simulink model "PrkgClimaEveMgr"  (R2022b)
settings: Solver=FixedStepDiscrete  StartTime=0.0  StopTime=10.0  FixedStep=0.2  RelTol=1e-3
(part 1 of 2)
settings Model: Creator=blue.ge  LastModifiedBy=blue.ge
settings DataIOCC: SaveFormat=StructureWithTime
settings OptimizationCC: DefaultParameterBehavior=Inlined  EnhancedBackFolding=on  PassReuseOutputArgsAs=Individual arguments  ZeroExternalMemoryAtStartup=off  ZeroInternalMemoryAtStartup=off  OptimizeBlockOrder=speed  DifferentSizesBufferReuse=on  OptimizationCustomize=off  EfficientTunableParamExpr=on
settings DebuggingCC: ParameterTunabilityLossMsg=error
settings ModelReferenceCC: ModelReferenceNumInstancesAllowed=Single
settings SFSimCC: MATLABDynamicMemAlloc=off
settings RTWCC: Description=AUTOSAR  SystemTargetFile=autosar.tlc  GenCodeOnly=on  TemplateMakefile=ert_default_tmf
settings CodeAppCC: ForceParamTrailComments=on  IgnoreCustomStorageClasses=off  OperatorAnnotations=on  SimulinkDataObjDesc=on  SFDataObjDesc=on  InsertBlockDesc=on
settings STFCustomTargetCC: TargetLangStandard=C99 (ISO)  MultiwordLength=256  ParMdlRefBuildCompliant=on  CompOptLevelCompliant=on  IncludeMdlTerminateFcn=off  CombineOutputUpdateFcns=on  SuppressErrorStatus=on  ERTCustomFileBanners=on  SupportNonFinite=off  SupportComplex=off  SupportContinuousTime=off  SupportNonInlinedSFcns=off  RemoveResetFunc=on  MATLABClassNameForMDSCustomization=Simulink.SoftwareTarget.ERTCustomization  AutosarCompliant=on  UseToolchainInfoCompliant=on  LookupTableObjectStructAxisOrder=2,1,3,4,...  LUTObjectStructOrderEvenSpacing=Size,Table,Breakpoints  SystemTargetFile=autosar.tlc
settings ERTTargetCC: TargetLangStandard=C99 (ISO)  ConcurrentExecutionCompliant=off  IncludeMdlTerminateFcn=off  SuppressErrorStatus=on  SupportNonFinite=off  SupportComplex=off  ModelStepFunctionPrototypeControlCompliant=off  CPPClassGenCompliant=off  AutosarCompliant=on  LookupTableObjectStructAxisOrder=2,1,3,4,...  LUTObjectStructOrderEvenSpacing=Size,Table,Breakpoints  InlinedParameterPlacement=NonHierarchical  ERTCustomFileTemplate=example_file_process.tlc

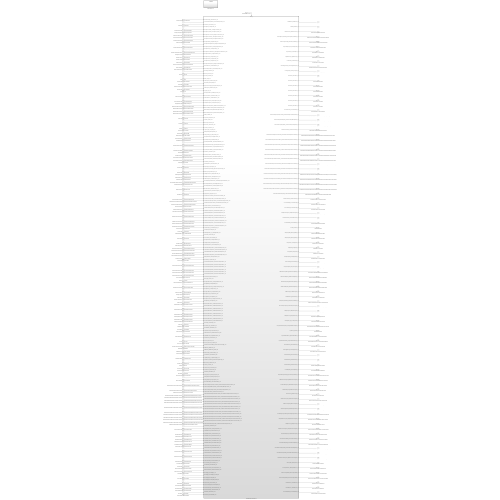
[thumbnail: Simulink's stored preview of the top-level diagram]
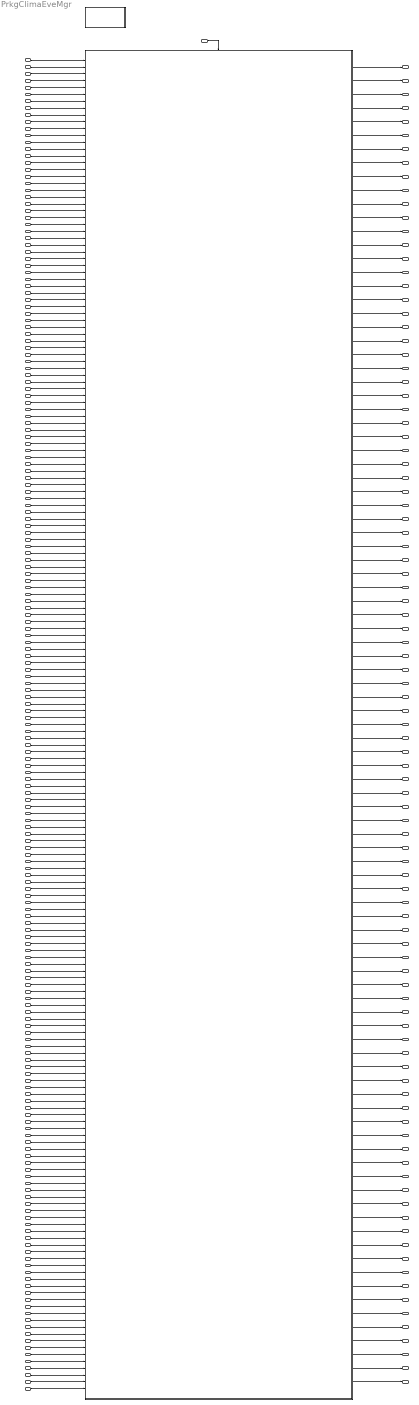
== system PrkgClimaEveMgr ==
  PrkgClimaEveMgr_Runnable : Inport  SampleTime=0.2  OutputFunctionCall=on
  AmbTFildForClimaNgcc_AmbTFildForClima : Inport  Port=2  OutDataTypeStr=T30  PortDimensions=1
  BookTrvlClimaActvReqfromExsrc_BookTrvlClimaActvReqfromExsrc : Inport  Port=3  OutDataTypeStr=Enum: OnOffNoReq  PortDimensions=1
  CarTiIntForClima_CarTiIntForClima : Inport  Port=4  OutDataTypeStr=CarTiGlb  PortDimensions=1
  ClimaCfgForCds1_ClimaCfgForCds1 : Inport  Port=5  OutDataTypeStr=Boolean  PortDimensions=1
  ClimaCfgForClimaCtrlMan1_ClimaCfgForClimaCtrlMan1 : Inport  Port=6  OutDataTypeStr=Boolean  PortDimensions=1
  ClimaCfgForCoolgPreCdn1_ClimaCfgForCoolgPreCdn1 : Inport  Port=7  OutDataTypeStr=Enum: ClimaCfgForCoolgPreCdn1  PortDimensions=1
  ClimaCfgForCooltCircForClima1_ClimaCfgForCooltCircForClima1 : Inport  Port=8  OutDataTypeStr=Boolean  PortDimensions=1
  ClimaCfgForEcuCooltVlvOutp1_ClimaCfgForEcuCooltVlvOutp1 : Inport  Port=9  OutDataTypeStr=Enum: ClimaCfgForEcuCooltVlvOutp1  PortDimensions=1
  ClimaCfgForExtVentnClimaCtrl_ClimaCfgForExtVentnClimaCtrl : Inport  Port=10  OutDataTypeStr=Enum: CarCfgForExtVentnClimaCtrl  PortDimensions=1
  ClimaCfgForFoldCSD_ClimaCfgForFoldCSD : Inport  Port=11  OutDataTypeStr=Boolean  PortDimensions=1
  ClimaCfgForForRemEngStrtPreCdn_ClimaCfgForForRemEngStrtPreCdn : Inport  Port=12  OutDataTypeStr=Enum: CarCfgForRemEngStrtPreCdn  PortDimensions=1
  ClimaCfgForHeatgPreCdnAvl1_ClimaCfgForHeatgPreCdnAvl1 : Inport  Port=13  OutDataTypeStr=Boolean  PortDimensions=1
  ClimaCfgForHeatrTyp1_ClimaCfgForHeatrTyp1 : Inport  Port=14  OutDataTypeStr=Enum: ClimaCfgForHeatrTyp1  PortDimensions=1
  ClimaCfgForHvacHeatrElecFrntPrsnt1_ClimaCfgForHvacHeatrElecFrntPrsnt1 : Inport  Port=15  OutDataTypeStr=Boolean  PortDimensions=1
  ClimaCfgForPostCdnAvl1_ClimaCfgForPostCdnAvl1 : Inport  Port=16  OutDataTypeStr=Boolean  PortDimensions=1
  ClimaCfgForPreClng1_ClimaCfgForPreClng1 : Inport  Port=17  OutDataTypeStr=Boolean  PortDimensions=1
  ClimaCfgForVehElec1_ClimaCfgForVehElec1 : Inport  Port=18  OutDataTypeStr=Boolean  PortDimensions=1
  ClimaCfgForVehHyb1_ClimaCfgForVehHyb1 : Inport  Port=19  OutDataTypeStr=Boolean  PortDimensions=1
  ClimaCfgForVrntOfVehSysElec1_ClimaCfgForVrntOfVehSysElec1 : Inport  Port=20  OutDataTypeStr=Enum: ClimaCenCfgForVrntOfVehSysElec1  PortDimensions=1
  ClimaOvrHeatProWarn_ClimaOvrHeatProWarn : Inport  Port=21  OutDataTypeStr=Enum: ClimaOvrheatProWarnSts  PortDimensions=1
  ClimaOvrHeatReqFromExrsc_ClimaOvrHeatReqFromExrsc : Inport  Port=22  OutDataTypeStr=Enum: PreCdngReqFromExtSrc  PortDimensions=1
  ClimaStrtTiDly_ClimaStrtTiDly : Inport  Port=23  OutDataTypeStr=Ti39  PortDimensions=1
  ClimaTmrSts_ClimaTmrSts : Inport  Port=24  OutDataTypeStr=Enum: OnOff1  PortDimensions=1
  CmptmtClsd_CmptmtClsd : Inport  Port=25  OutDataTypeStr=Boolean  PortDimensions=1
  COHSts_COHSts : Inport  Port=26  OutDataTypeStr=Enum: CabinOHSts  PortDimensions=1
  CooltTForPrkgClima_CooltTForPrkgClima : Inport  Port=27  OutDataTypeStr=T30  PortDimensions=1
  DCChrgnHndlSts_DCChrgnHndlSts : Inport  Port=28  OutDataTypeStr=Enum: DCChrgnHndlStsE2E  PortDimensions=1
  DlyReqForTmrForPrkgClima_DlyReqForTmrForPrkgClima : Inport  Port=29  OutDataTypeStr=Boolean  PortDimensions=1
  DshbTForPrkgClima_DshbTForPrkgClima : Inport  Port=30  OutDataTypeStr=T30  PortDimensions=1
  EngT_Ngcc_EngT : Inport  Port=31  OutDataTypeStr=Bus: EngT  PortDimensions=1  BusOutputAsStruct=on
  ErsCmdByKeyFromExsrc_ErsCmdByKeyFromExsrc : Inport  Port=32  OutDataTypeStr=Enum: ErsCmd  PortDimensions=1
  ErsRunTimeFromExtSrc_ErsRunTimeFromExtSrc : Inport  Port=33  OutDataTypeStr=Ti39  PortDimensions=1
  HeatrElecNotAvlForTmr_HeatrElecNotAvlForTmr : Inport  Port=34  OutDataTypeStr=Boolean  PortDimensions=1
  HeatrTypSetgForPrkgClima_HeatrTypSetgForPrkgClima : Inport  Port=35  OutDataTypeStr=Enum: HeatrPreCdngTyp  PortDimensions=1
  HeatUpRqrdForPrkgClima_HeatUpRqrdForPrkgClima : Inport  Port=36  OutDataTypeStr=Boolean  PortDimensions=1
  HMIExtVentFanVolReqFromExtSrc_HMIExtVentFanVolReqFromExtSrc : Inport  Port=37  OutDataTypeStr=Enum: FanVolReq  PortDimensions=1
  HMIExtVentonoffReqFromExtSrc_HMIExtVentonoffReqFromExtSrc : Inport  Port=38  OutDataTypeStr=Enum: OnOffNoReq  PortDimensions=1
  HMIExtVentTSpReqFromExtSrc_HMIExtVentTSpReqFromExtSrc : Inport  Port=39  OutDataTypeStr=Enum: TSpReq  PortDimensions=1
  HMIExtVentTStsReqFromExtSrc_HMIExtVentTStsReqFromExtSrc : Inport  Port=40  OutMin=-256  OutMax=255.9  OutDataTypeStr=T2  PortDimensions=1
  HvBattSoc_HvBattSoc : Inport  Port=41  OutDataTypeStr=Perc7  PortDimensions=1
  HvSysCnctWoU_HvSysCnctWoU : Inport  Port=42  OutDataTypeStr=Boolean  PortDimensions=1
  HvSysWoU_HvSysWoU : Inport  Port=43  OutDataTypeStr=Boolean  PortDimensions=1
  IntCo2ActvSts_IntCo2ActvSts : Inport  Port=44  OutDataTypeStr=Enum: IntCo2Sts  PortDimensions=1
  IntCo2fromExsrc_IntCo2fromExsrc : Inport  Port=45  OutDataTypeStr=Enum: OnOff1  PortDimensions=1
  KeyClimaActv_KeyClimaActv : Inport  Port=46  OutDataTypeStr=Enum: OnOff1  PortDimensions=1
  LockgCenSts_Ngcc_LockgCenSts : Inport  Port=47  OutDataTypeStr=Bus: LockgCenSts3  PortDimensions=1  BusOutputAsStruct=on
  OnBdChrgrHndlSts_OnBdChrgrHndlSts : Inport  Port=48  OutDataTypeStr=Enum: OnBdChrgrSts1  PortDimensions=1
  PostDrvgClimaReq_Ngcc_PostDrvgClimaReq : Inport  Port=49  OutDataTypeStr=Enum: OnOff1  PortDimensions=1
  PreClngRqrdFromExtSrc_PreClngRqrdFromExtSrc : Inport  Port=50  OutDataTypeStr=Enum: PreCdngReqFromExtSrc  PortDimensions=1
  PrkgClimaDiByCarMod_PrkgClimaDiByCarMod : Inport  Port=51  OutDataTypeStr=Boolean  PortDimensions=1
  PrkgClimaRqrdImdtForBattULo_PrkgClimaRqrdImdtForBattULo : Inport  Port=52  OutDataTypeStr=Boolean  PortDimensions=1
  PrkgClimaRqrdImdtFromActvMgr_PrkgClimaRqrdImdtFromActvMgr : Inport  Port=53  OutDataTypeStr=Boolean  PortDimensions=1
  PrkgClimaRqrdImdtFromExtSrc_PrkgClimaRqrdImdtFromExtSrc : Inport  Port=54  OutDataTypeStr=Enum: PreCdngReqFromExtSrc  PortDimensions=1
  PrkgClimaRqrdTmrForBattULo_PrkgClimaRqrdTmrForBattULo : Inport  Port=55  OutDataTypeStr=Boolean  PortDimensions=1
  RemClimaFnshd_RemClimaFnshd : Inport  Port=56  OutDataTypeStr=Boolean  PortDimensions=1
  RemClimaReqFromExtSrc_RemClimaReqFromExtSrc : Inport  Port=57  OutDataTypeStr=Enum: OnOffNoReq  PortDimensions=1
  RemStrtExtnTiReqFromExtSrc_RemStrtExtnTiReqFromExtSrc : Inport  Port=58  OutDataTypeStr=Ti39  PortDimensions=1
  RemStrtHvCtrlReqFromExtSrc_RemStrtHvCtrlReqFromExtSrc : Inport  Port=59  OutDataTypeStr=Enum: ErsCmd  PortDimensions=1
  RemVentFbSts_RemVentFbSts : Inport  Port=60  OutDataTypeStr=Enum: RemVentSts  PortDimensions=1
  RemVentReqfromExsrc_RemVentReqfromExsrc : Inport  Port=61  OutDataTypeStr=Enum: OnOffNoReq  PortDimensions=1
  ReqForPrkgClima_ReqForPrkgClima : Inport  Port=62  OutDataTypeStr=Enum: PrkgClimaReqSts  PortDimensions=1
  ReqForSwtHeatrTypFromActvMgr_ReqForSwtHeatrTypFromActvMgr : Inport  Port=63  OutDataTypeStr=Boolean  PortDimensions=1
  RtSdlForPrkgClima_RtSdlForPrkgClima : Inport  Port=64  OutDataTypeStr=Ti33  PortDimensions=1
  SeatHeatgEnadFromHmi_SeatHeatgEnadFromHmi : Inport  Port=65  OutDataTypeStr=Enum: SeatHeatDurgClimaEnad  PortDimensions=1
  SeatHeatgEnadFromTelm_SeatHeatgEnadFromTelm : Inport  Port=66  OutDataTypeStr=Enum: SeatHeatDurgClimaEnad  PortDimensions=1
  SnsrQfOkForPrkgClima_SnsrQfOkForPrkgClima : Inport  Port=67  OutDataTypeStr=Boolean  PortDimensions=1
  SteerWhlHeatgDurgClimaEnadFromExsrc_SteerWhlHeatgDurgClimaEnadFromExsrc : Inport  Port=68  OutDataTypeStr=Enum: OnOffNoReq  PortDimensions=1
  SteerWhlHeatgEnadFromHmi_SteerWhlHeatgEnadFromHmi : Inport  Port=69  OutDataTypeStr=Boolean  PortDimensions=1
  SteerWhlHeatgEnadFromTelm_SteerWhlHeatgEnadFromTelm : Inport  Port=70  OutDataTypeStr=Boolean  PortDimensions=1
  StrtRemStsFromExsrc_StrtRemStsFromExsrc : Inport  Port=71  OutDataTypeStr=Enum: StrtRemSts1  PortDimensions=1
  SuspStrtActvForPrkgClima_SuspStrtActvForPrkgClima : Inport  Port=72  OutDataTypeStr=Boolean  PortDimensions=1
  SysDogSlpCountTi_SysDogSlpCountTi : Inport  Port=73  OutDataTypeStr=Ti33  PortDimensions=1
  TelmClimaExtVentnLvlFromExtSrc_TelmClimaExtVentnLvlFromExtSrc : Inport  Port=74  OutDataTypeStr=Enum: HmiHvacFanLvl  PortDimensions=1
  TelmClimaExtVentnReqFromExtSrc_TelmClimaExtVentnReqFromExtSrc : Inport  Port=75  OutDataTypeStr=Enum: OnOffNoReq  PortDimensions=1
  TelmClimaExtVentnTempRangeFromExtSrc_TelmClimaExtVentnTempRangeFromExtSrc : Inport  Port=76  OutMin=-256  OutMax=255.9  OutDataTypeStr=T2  PortDimensions=1
  TelmClimaExtVentnTSpSpclFromExtSrc_TelmClimaExtVentnTSpSpclFromExtSrc : Inport  Port=77  OutDataTypeStr=Enum: HmiCmptmtTSpSpcl  PortDimensions=1
  TelmClimaTmrFromExtSrc_TelmClimaTmrFromExtSrc : Inport  Port=78  OutDataTypeStr=Ti33  PortDimensions=1
  TelmSeatDrvrHeatReqFromExsrc_TelmSeatDrvrHeatReqFromExsrc : Inport  Port=79  OutDataTypeStr=Enum: TelmSeatClimaLvlExsrc  PortDimensions=1
  TelmSeatDrvVentnReqFromExsrc_TelmSeatDrvVentnReqFromExsrc : Inport  Port=80  OutDataTypeStr=Enum: TelmSeatClimaLvlExsrc  PortDimensions=1
  TelmSeatPassHeatReqFromExsrc_TelmSeatPassHeatReqFromExsrc : Inport  Port=81  OutDataTypeStr=Enum: TelmSeatClimaLvlExsrc  PortDimensions=1
  TelmSeatPassVentnReqFromExsrc_TelmSeatPassVentnReqFromExsrc : Inport  Port=82  OutDataTypeStr=Enum: TelmSeatClimaLvlExsrc  PortDimensions=1
  TelmSeatSecLeHeatReqFromExsrc_TelmSeatSecLeHeatReqFromExsrc : Inport  Port=83  OutDataTypeStr=Enum: TelmSeatClimaLvlExsrc  PortDimensions=1
  TelmSeatSecLeVentnReqFromExsrc_TelmSeatSecLeVentnReqFromExsrc : Inport  Port=84  OutDataTypeStr=Enum: TelmSeatClimaLvlExsrc  PortDimensions=1
  TelmSeatSecRiHeatReqFromExsrc_TelmSeatSecRiHeatReqFromExsrc : Inport  Port=85  OutDataTypeStr=Enum: TelmSeatClimaLvlExsrc  PortDimensions=1
  TelmSeatSecRiVentnReqFromExsrc_TelmSeatSecRiVentnReqFromExsrc : Inport  Port=86  OutDataTypeStr=Enum: TelmSeatClimaLvlExsrc  PortDimensions=1
  TForHeatrPreCdngTyp_TForHeatrPreCdngTyp : Inport  Port=87  OutDataTypeStr=T3  PortDimensions=1
  TmrForPrkgClima_TmrForPrkgClima : Inport  Port=88  OutDataTypeStr=CarTiGlb  PortDimensions=1
  VehModMngtGlbSafe1_Ngcc_VehModMngtGlbSafe1 : Inport  Port=89  OutDataTypeStr=Bus: VehModMngtGlbSafe1  PortDimensions=1  BusOutputAsStruct=on
  VehSunLoadTot_VehSunLoadTot : Inport  Port=90  OutDataTypeStr=SunLoad30  PortDimensions=1
  VehSunLoadTotFild_VehSunLoadTotFild : Inport  Port=91  OutDataTypeStr=SunLoad30  PortDimensions=1
  ClimaCfgForStatrtDevice_ClimaCfgForStartDevice : Inport  Port=92  OutDataTypeStr=Enum: StartDevice  PortDimensions=1
  PostHeatgRunTiEstimd_PostHeatgRunTiEstimd : Inport  Port=93  OutDataTypeStr=UInt16  PortDimensions=1
  TelmDefrostReqFromExsrc_TelmDefrostReqFromExsrc : Inport  Port=94  OutDataTypeStr=Enum: OnOffNoReq  PortDimensions=1
  TelmSeatThrdLeHeatReqFromExsrc_TelmSeatThrdLeHeatReqFromExsrc : Inport  Port=95  OutDataTypeStr=Enum: TelmSeatClimaLvlExsrc  PortDimensions=1
  TelmSeatThrdLeVentnReqFromExsrc_TelmSeatThrdLeVentnReqFromExsrc : Inport  Port=96  OutDataTypeStr=Enum: TelmSeatClimaLvlExsrc  PortDimensions=1
  TelmSeatThrdRiHeatReqFromExsrc_TelmSeatThrdRiHeatReqFromExsrc : Inport  Port=97  OutDataTypeStr=Enum: TelmSeatClimaLvlExsrc  PortDimensions=1
  TelmSeatThrdRiVentnReqFromExsrc_TelmSeatThrdRiVentnReqFromExsrc : Inport  Port=98  OutDataTypeStr=Enum: TelmSeatClimaLvlExsrc  PortDimensions=1
  TempRangeFromExtSrc_TempRangeFromExtSrc : Inport  Port=99  OutMin=-256  OutMax=255.9  OutDataTypeStr=T2  PortDimensions=1
  CmptmtTFrntRaw_CmptmtTFrntRaw : Inport  Port=100  OutDataTypeStr=Bus: CmptmtTFrnt  PortDimensions=1  BusOutputAsStruct=on
  BookTrvlClimaActvReq2FromExSrc_BookTrvlClimaActvReq2FromExSrc : Inport  Port=101  OutDataTypeStr=Enum: OnOffNoReq  PortDimensions=1
  BookTrvlClimaActvReq3FromExSrc_BookTrvlClimaActvReq3FromExSrc : Inport  Port=102  OutDataTypeStr=Enum: OnOffNoReq  PortDimensions=1
  BookTrvlClimaActvReq4FromExSrc_BookTrvlClimaActvReq4FromExSrc : Inport  Port=103  OutDataTypeStr=Enum: OnOffNoReq  PortDimensions=1
  BookTrvlClimaActvReq5FromExSrc_BookTrvlClimaActvReq5FromExSrc : Inport  Port=104  OutDataTypeStr=Enum: OnOffNoReq  PortDimensions=1
  BookTrvlClimaActvReq6FromExSrc_BookTrvlClimaActvReq6FromExSrc : Inport  Port=105  OutDataTypeStr=Enum: OnOffNoReq  PortDimensions=1
  BookTrvlClimaActvReq7FromExSrc_BookTrvlClimaActvReq7FromExSrc : Inport  Port=106  OutDataTypeStr=Enum: OnOffNoReq  PortDimensions=1
  BookTrvlClimaFnshd_BookTrvlClimaFnshd : Inport  Port=107  OutDataTypeStr=Boolean  PortDimensions=1
  ClimaActv_Ngcc_ClimaActv : Inport  Port=108  OutDataTypeStr=Enum: OnOff1  PortDimensions=1
  ClimaCfgForAirMtrlSnsrPrsnt1_ClimaCfgForAirMtrlSnsrPrsnt1 : Inport  Port=109  OutDataTypeStr=Enum: CarCfgForAirMtrlSnsrGly  PortDimensions=1
  ClimaCfgForBRAND_ClimaCfgForBRAND : Inport  Port=110  OutDataTypeStr=Enum: ClimaCfgForBRAND  PortDimensions=1
  ClimaCfgForCabinOxgenSupply_ClimaCfgForCabinOxgenSupply : Inport  Port=111  OutDataTypeStr=Enum: CabinOxgenSupply  PortDimensions=1
  ClimaCfgForHvacTyp_ClimaCfgForHvacTyp : Inport  Port=112  OutDataTypeStr=Enum: CarCfgForHvacTyp2  PortDimensions=1
  ClimaCfgForLifeDetection_ClimaCfgForLifeDetection : Inport  Port=113  OutDataTypeStr=Enum: LifeDetection  PortDimensions=1
  ClimaCfgForPrpsnTyp_ClimaCfgForPrpsnTyp : Inport  Port=114  OutDataTypeStr=Enum: CarCfgForPrpsnTypGly  PortDimensions=1
  ClimaCfgForRFKEY_ClimaCfgForRFKEY : Inport  Port=115  OutDataTypeStr=Enum: RFKEY  PortDimensions=1
  ClimaCfgForSnsrHumPrsnt1_ClimaCfgForSnsrHumPrsnt1 : Inport  Port=116  OutDataTypeStr=Boolean  PortDimensions=1
  ClimaCfgForVehTyp_ClimaCfgForVehTyp : Inport  Port=117  OutDataTypeStr=Enum: CarCfgForVehTyp3  PortDimensions=1
  ClimaWakUpTiDif1FromExSrc_ClimaWakUpTiDif1FromExSrc : Inport  Port=118  OutDataTypeStr=Mins2  PortDimensions=1
  ClimaWakUpTiDif2FromExSrc_ClimaWakUpTiDif2FromExSrc : Inport  Port=119  OutDataTypeStr=Mins2  PortDimensions=1
  ClimaWakUpTiDif3FromExSrc_ClimaWakUpTiDif3FromExSrc : Inport  Port=120  OutDataTypeStr=Mins2  PortDimensions=1
  ClimaWakUpTiDif4FromExSrc_ClimaWakUpTiDif4FromExSrc : Inport  Port=121  OutDataTypeStr=Mins2  PortDimensions=1
  ClimaWakUpTiDif5FromExSrc_ClimaWakUpTiDif5FromExSrc : Inport  Port=122  OutDataTypeStr=Mins2  PortDimensions=1
  ClimaWakUpTiDif6FromExSrc_ClimaWakUpTiDif6FromExSrc : Inport  Port=123  OutDataTypeStr=Mins2  PortDimensions=1
  ClimaWakUpTiDif7FromExSrc_ClimaWakUpTiDif7FromExSrc : Inport  Port=124  OutDataTypeStr=Mins2  PortDimensions=1
  CmptmtAirTEstimdAtRowFirst_CmptmtAirTEstimdAtRowFirst : Inport  Port=125  OutDataTypeStr=T30  PortDimensions=1
  CmptmtClngActv_CmptmtClngActv : Inport  Port=126  OutDataTypeStr=Enum: CmptmtClngActv  PortDimensions=1
  CmptmtRelHum_Ngcc_CmptmtRelHum : Inport  Port=127  OutDataTypeStr=Bus: CmptmRelHum  PortDimensions=1  BusOutputAsStruct=on
  CmptmtTRearRaw_CmptmtTRearRaw : Inport  Port=128  OutDataTypeStr=Bus: CmptmtTFrnt  PortDimensions=1  BusOutputAsStruct=on
  DoorOpenStsForClima_DoorOpenStsForClima : Inport  Port=129  OutDataTypeStr=Boolean  PortDimensions=1
  DoorStsWithOutTrForClima_DoorStsWithOutTrForClima : Inport  Port=130  OutDataTypeStr=Boolean  PortDimensions=1
  EngSt1WdStsFromExsrc_EngSt1WdStsFromExsrc : Inport  Port=131  OutDataTypeStr=Enum: EngSt1  PortDimensions=1
  FrntHvacBlowerSts_FrntHvacBlowerSts : Inport  Port=132  OutDataTypeStr=Enum: HvacFanSts  PortDimensions=1
  GearLvrIndcn_GearLvrIndcn : Inport  Port=133  OutDataTypeStr=Enum: GearLvrIndcn2  PortDimensions=1
  GearLvrIndcn_GearLvrIndcn_IsUpdated : Inport  Port=134  OutDataTypeStr=boolean  PortDimensions=1
  HmiWinterLockCarPrevnIceSetNgcc_HmiWinterLockCarPrevnIceSetNgcc : Inport  Port=135  OutDataTypeStr=Enum: OnOff1  PortDimensions=1
  HvBattMntnReq_HvBattMntnReq : Inport  Port=136  OutDataTypeStr=Enum: HvBattMntnReq  PortDimensions=1
  HvBattMntnReq_HvBattMntnReq_IsUpdated : Inport  Port=137  OutDataTypeStr=boolean  PortDimensions=1
  InflateAirSrcSwtNgcc_InflateAirSrcSwtNgcc : Inport  Port=138  OutDataTypeStr=Enum: OnOffNoReq  PortDimensions=1
  KeyClimaActvFnshd_KeyClimaActvFnshd : Inport  Port=139  OutDataTypeStr=Boolean  PortDimensions=1
  LonglifeBatteryReqNgcc_LonglifeBatteryReqNgcc : Inport  Port=140  OutDataTypeStr=Enum: BatteryReq  PortDimensions=1
  MemReadForPrkgClimaEveMgr_MemReadForPrkgClimaEveMgr : Inport  Port=141  OutDataTypeStr=Bus: MemRecForPrkgClimaEveMgr  PortDimensions=1  BusOutputAsStruct=on
  OGMAirSrcFnshd_OGMAirSrcFnshd : Inport  Port=142  OutDataTypeStr=Boolean  PortDimensions=1
  PrvntWinFrz_PrvntWinFrz : Inport  Port=143  OutDataTypeStr=Boolean  PortDimensions=1
  PrvntWinFrzFnshd_PrvntWinFrzFnshd : Inport  Port=144  OutDataTypeStr=Boolean  PortDimensions=1
  RemClimaFnshd1_RemClimaFnshd1 : Inport  Port=145  OutDataTypeStr=Boolean  PortDimensions=1
  RemClimaWarn_RemClimaWarn : Inport  Port=146  OutDataTypeStr=Enum: ClimaWarn  PortDimensions=1
  RemOxygenSplyFnshd_RemOxygenSplyFnshd : Inport  Port=147  OutDataTypeStr=Boolean  PortDimensions=1
  RemOxygenSplyFnshd1_RemOxygenSplyFnshd1 : Inport  Port=148  OutDataTypeStr=Boolean  PortDimensions=1
  RemOxygenSplyWarn_RemOxygenSplyWarn : Inport  Port=149  OutDataTypeStr=Enum: ClimaWarn  PortDimensions=1
  TcsOvrheatdProtnStsNgcc_TcsOvrheatdProtnStsNgcc : Inport  Port=150  OutMin=0  OutMax=3  OutDataTypeStr=UInt2  PortDimensions=1
  TelmBookClimaReqHmiCmptmtTSpSpclFromExtSrc_TelmBookClimaReqHmiCmptmtTSpSpclFromExtSrc : Inport  Port=151  OutDataTypeStr=Enum: NormalLowHigh  PortDimensions=1
  TelmBookClimaReqOnOffNoReqFromExtSrc_TelmBookClimaReqOnOffNoReqFromExtSrc : Inport  Port=152  OutDataTypeStr=Enum: NoReqOnOff  PortDimensions=1
  TelmBookClimaReqTempRangeFromExtSrc_TelmBookClimaReqTempRangeFromExtSrc : Inport  Port=153  OutDataTypeStr=TempRange  PortDimensions=1
  TelmBookClimaTmrFromExtSrc_TelmBookClimaTmrFromExtSrc : Inport  Port=154  OutDataTypeStr=Ti33  PortDimensions=1
  TelmBookSeatHeatClimalReqDrvHeatClimaLvlFromExtSrc_TelmBookSeatHeatClimalReqDrvHeatClimaLvlFromExtSrc : Inport  Port=155  OutDataTypeStr=Enum: TelmSeatClimaLvlExsrc  PortDimensions=1
  TelmBookSeatHeatClimalReqPassHeatClimaLvlFromExtSrc_TelmBookSeatHeatClimalReqPassHeatClimaLvlFromExtSrc : Inport  Port=156  OutDataTypeStr=Enum: TelmSeatClimaLvlExsrc  PortDimensions=1
  TelmBookSeatHeatClimalReqSecLeHeatClimaLvlFromExtSrc_TelmBookSeatHeatClimalReqSecLeHeatClimaLvlFromExtSrc : Inport  Port=157  OutDataTypeStr=Enum: TelmSeatClimaLvlExsrc  PortDimensions=1
  TelmBookSeatHeatClimalReqSecRiHeatClimaLvlFromExtSrc_TelmBookSeatHeatClimalReqSecRiHeatClimaLvlFromExtSrc : Inport  Port=158  OutDataTypeStr=Enum: TelmSeatClimaLvlExsrc  PortDimensions=1
  TelmBookSeatHeatClimalReqThrdLeHeatClimaLvlFromExtSrc_TelmBookSeatHeatClimalReqThrdLeHeatClimaLvlFromExtSrc : Inport  Port=159  OutDataTypeStr=Enum: TelmSeatClimaLvlExsrc  PortDimensions=1
  TelmBookSeatHeatClimalReqThrdRiHeatClimaLvlFromExtSrc_TelmBookSeatHeatClimalReqThrdRiHeatClimaLvlFromExtSrc : Inport  Port=160  OutDataTypeStr=Enum: TelmSeatClimaLvlExsrc  PortDimensions=1
  TelmBookSeatVentnClimalReqDrvVentnClimaLvlFromExtSrc_TelmBookSeatVentnClimalReqDrvVentnClimaLvlFromExtSrc : Inport  Port=161  OutDataTypeStr=Enum: TelmSeatClimaLvlExsrc  PortDimensions=1
  TelmBookSeatVentnClimalReqPassVentnClimaLvlFromExtSrc_TelmBookSeatVentnClimalReqPassVentnClimaLvlFromExtSrc : Inport  Port=162  OutDataTypeStr=Enum: TelmSeatClimaLvlExsrc  PortDimensions=1
  TelmBookSeatVentnClimalReqSecLeVentnClimaLvlFromExtSrc_TelmBookSeatVentnClimalReqSecLeVentnClimaLvlFromExtSrc : Inport  Port=163  OutDataTypeStr=Enum: TelmSeatClimaLvlExsrc  PortDimensions=1
  TelmBookSeatVentnClimalReqSecRiVentnClimaLvlFromExtSrc_TelmBookSeatVentnClimalReqSecRiVentnClimaLvlFromExtSrc : Inport  Port=164  OutDataTypeStr=Enum: TelmSeatClimaLvlExsrc  PortDimensions=1
  TelmBookSeatVentnClimalReqThrdLeVentnClimaLvlFromExtSrc_TelmBookSeatVentnClimalReqThrdLeVentnClimaLvlFromExtSrc : Inport  Port=165  OutDataTypeStr=Enum: TelmSeatClimaLvlExsrc  PortDimensions=1
  TelmBookSeatVentnClimalReqThrdRiVentnClimaLvlFromExtSrc_TelmBookSeatVentnClimalReqThrdRiVentnClimaLvlFromExtSrc : Inport  Port=166  OutDataTypeStr=Enum: TelmSeatClimaLvlExsrc  PortDimensions=1
  TelmBookSteerWhlHeatClimalReqFromExtSrc_TelmBookSteerWhlHeatClimalReqFromExtSrc : Inport  Port=167  OutDataTypeStr=Enum: OnOffNoReq  PortDimensions=1
  TelmDefrostFnshd_TelmDefrostFnshd : Inport  Port=168  OutDataTypeStr=Boolean  PortDimensions=1
  TelmOxygenReqFromExSrc_TelmOxygenReqFromExSrc : Inport  Port=169  OutDataTypeStr=Enum: OnOffNoReq  PortDimensions=1
  TelmSeatHeatDrvrFnshd_TelmSeatHeatDrvrFnshd : Inport  Port=170  OutDataTypeStr=Boolean  PortDimensions=1
  TelmSeatHeatPassFnshd_TelmSeatHeatPassFnshd : Inport  Port=171  OutDataTypeStr=Boolean  PortDimensions=1
  TelmSeatHeatSecLeFnshd_TelmSeatHeatSecLeFnshd : Inport  Port=172  OutDataTypeStr=Boolean  PortDimensions=1
  TelmSeatHeatSecRiFnshd_TelmSeatHeatSecRiFnshd : Inport  Port=173  OutDataTypeStr=Boolean  PortDimensions=1
  TelmSeatHeatThrdLeFnshd_TelmSeatHeatThrdLeFnshd : Inport  Port=174  OutDataTypeStr=Boolean  PortDimensions=1
  TelmSeatHeatThrdRiFnshd_TelmSeatHeatThrdRiFnshd : Inport  Port=175  OutDataTypeStr=Boolean  PortDimensions=1
  TelmSeatVentnDrvrFnshd_TelmSeatVentnDrvrFnshd : Inport  Port=176  OutDataTypeStr=Boolean  PortDimensions=1
  TelmSeatVentnPassFnshd_TelmSeatVentnPassFnshd : Inport  Port=177  OutDataTypeStr=Boolean  PortDimensions=1
  TelmSeatVentnSecLeFnshd_TelmSeatVentnSecLeFnshd : Inport  Port=178  OutDataTypeStr=Boolean  PortDimensions=1
  TelmSeatVentnSecRiFnshd_TelmSeatVentnSecRiFnshd : Inport  Port=179  OutDataTypeStr=Boolean  PortDimensions=1
  TelmSeatVentnThrdLeFnshd_TelmSeatVentnThrdLeFnshd : Inport  Port=180  OutDataTypeStr=Boolean  PortDimensions=1
  TelmSeatVentnThrdRiFnshd_TelmSeatVentnThrdRiFnshd : Inport  Port=181  OutDataTypeStr=Boolean  PortDimensions=1
  TelmSteerWhlHeatFnshd_TelmSteerWhlHeatFnshd : Inport  Port=182  OutDataTypeStr=Boolean  PortDimensions=1
  UnLckClimaActvReq_UnLckClimaActvReq : Inport  Port=183  OutDataTypeStr=Enum: UnLckCoolActvLvl  PortDimensions=1
  UnLckHeatActvReq_UnLckHeatActvReq : Inport  Port=184  OutDataTypeStr=Enum: UnLckHeatActvLvl  PortDimensions=1
  VentnForWinOpenGlbActv_VentnForWinOpenGlbActv : Inport  Port=185  OutDataTypeStr=Boolean  PortDimensions=1
  VTMOverTProtectionFnshd_VTMOverTProtectionFnshd : Inport  Port=186  OutDataTypeStr=Boolean  PortDimensions=1
  WarnLvlMsgNgcc_WarnLvlMsgNgcc : Inport  Port=187  OutDataTypeStr=Enum: WarnLvl  PortDimensions=1
  WarnLvlMsgNgcc_WarnLvlMsgNgcc_IsUpdated : Inport  Port=188  OutDataTypeStr=boolean  PortDimensions=1
  WarnLvlMsgUWB_WarnLvlMsgUWB : Inport  Port=189  OutDataTypeStr=Enum: WarnLvl  PortDimensions=1
  WarnLvlMsgUWB_WarnLvlMsgUWB_IsUpdated : Inport  Port=190  OutDataTypeStr=boolean  PortDimensions=1
  WinOpenLDACReq_WinOpenLDACReq : Inport  Port=191  OutDataTypeStr=Enum: OnOff1  PortDimensions=1
  WinOpenLDACReqUWB_WinOpenLDACReqUWB : Inport  Port=192  OutDataTypeStr=Enum: OnOff1  PortDimensions=1
  WinPosnStsAtDrvrReNgcc_WinPosnStsAtDrvrReNgcc : Inport  Port=193  OutDataTypeStr=Enum: WinAndRoofAndCurtPosnTyp  PortDimensions=1
  WinPosnStsAtPassReNgcc_WinPosnStsAtPassRe : Inport  Port=194  OutDataTypeStr=Enum: WinAndRoofAndCurtPosnTyp  PortDimensions=1
  WinPosnStsDrv_WinPosnStsAtDrv : Inport  Port=195  OutDataTypeStr=Enum: WinAndRoofAndCurtPosnTyp  PortDimensions=1
  WinPosnStsPass_WinPosnStsAtPass : Inport  Port=196  OutDataTypeStr=Enum: WinAndRoofAndCurtPosnTyp  PortDimensions=1
  PrkgClimaEveMgr_Init : Subsystem
  PrkgClimaEveMgr_Runnable_sys : Subsystem
  HvBattMntnActv_HvBattMntnActv : Outport  OutDataTypeStr=Boolean  PortDimensions=1
  LDACState_LDACState : Outport  Port=2  OutDataTypeStr=Boolean  PortDimensions=1
  MemBookTrvlClimaTi_MemBookTrvlClimaTi : Outport  Port=3  OutDataTypeStr=Mins2  PortDimensions=1
  MemSaveFlgForPrkgClimaEveMgr_MemSaveFlgForPrkgClimaEveMgr : Outport  Port=4  OutDataTypeStr=Boolean  PortDimensions=1
  MemWrForPrkgClimaEveMgr_MemWrForPrkgClimaEveMgr : Outport  Port=5  OutDataTypeStr=Bus: MemRecForPrkgClimaEveMgr  PortDimensions=1  BusOutputAsStruct=on
  PrkgClimaReqExcpSeat_PrkgClimaReqExcpSeat : Outport  Port=6  OutDataTypeStr=Boolean  PortDimensions=1
  PrkgClimaReqPrio_PrkgClimaReqPrio : Outport  Port=7  OutDataTypeStr=Boolean  PortDimensions=1
  PrkgOGMAirSrcReq_PrkgOGMAirSrcReq : Outport  Port=8  OutDataTypeStr=Boolean  PortDimensions=1
  PrvntWinActvReq_PrvntWinActvReq : Outport  Port=9  OutDataTypeStr=Boolean  PortDimensions=1
  RemClimaEgyExsrcReqPlus_RemClimaEgyExsrcReqPlus : Outport  Port=10  OutDataTypeStr=UInt32  PortDimensions=1
  RemOxygenSplyReq_RemOxygenSplyReq : Outport  Port=11  OutDataTypeStr=Boolean  PortDimensions=1
  TcamClcltTiFb1_TcamClcltTiFb1 : Outport  Port=12  OutDataTypeStr=Mins2  PortDimensions=1
  TcamClcltTiFb2_TcamClcltTiFb2 : Outport  Port=13  OutDataTypeStr=Mins2  PortDimensions=1
  TcamClcltTiFb3_TcamClcltTiFb3 : Outport  Port=14  OutDataTypeStr=Mins2  PortDimensions=1
  TcamClcltTiFb4_TcamClcltTiFb4 : Outport  Port=15  OutDataTypeStr=Mins2  PortDimensions=1
  TcamClcltTiFb5_TcamClcltTiFb5 : Outport  Port=16  OutDataTypeStr=Mins2  PortDimensions=1
  TcamClcltTiFb6_TcamClcltTiFb6 : Outport  Port=17  OutDataTypeStr=Mins2  PortDimensions=1
  TcamClcltTiFb7_TcamClcltTiFb7 : Outport  Port=18  OutDataTypeStr=Mins2  PortDimensions=1
  TcsOvrheatdProtnReq_TcsOvrheatdProtnReq : Outport  Port=19  OutDataTypeStr=Boolean  PortDimensions=1
  TelmBookClimaReqHmiCmptmtTSpSpclStrtReq_TelmBookClimaReqHmiCmptmtTSpSpclStrtReq : Outport  Port=20  OutDataTypeStr=Enum: NormalLowHigh  PortDimensions=1
  TelmBookClimaReqOnOffNoReqStrtReq_TelmBookClimaReqOnOffNoReqStrtReq : Outport  Port=21  OutDataTypeStr=Enum: NoReqOnOff  PortDimensions=1
  TelmBookClimaReqTempRangeStrtReq_TelmBookClimaReqTempRangeStrtReq : Outport  Port=22  OutDataTypeStr=TempRange  PortDimensions=1
  TelmBookClimaTmrStrtReq_TelmBookClimaTmrStrtReq : Outport  Port=23  OutDataTypeStr=Ti33  PortDimensions=1
  TelmBookSeatHeatClimalReqDrvHeatClimaLvlStrtReq_TelmBookSeatHeatClimalReqDrvHeatClimaLvlStrtReq : Outport  Port=24  OutDataTypeStr=Enum: TelmSeatClimaLvl  PortDimensions=1
  TelmBookSeatHeatClimalReqPassHeatClimaLvlStrtReq_TelmBookSeatHeatClimalReqPassHeatClimaLvlStrtReq : Outport  Port=25  OutDataTypeStr=Enum: TelmSeatClimaLvl  PortDimensions=1
  TelmBookSeatHeatClimalReqSecLeHeatClimaLvlStrtReq_TelmBookSeatHeatClimalReqSecLeHeatClimaLvlStrtReq : Outport  Port=26  OutDataTypeStr=Enum: TelmSeatClimaLvl  PortDimensions=1
  TelmBookSeatHeatClimalReqSecRiHeatClimaLvlStrtReq_TelmBookSeatHeatClimalReqSecRiHeatClimaLvlStrtReq : Outport  Port=27  OutDataTypeStr=Enum: TelmSeatClimaLvl  PortDimensions=1
  TelmBookSeatHeatClimalReqThrdLeHeatClimaLvlStrtReq_TelmBookSeatHeatClimalReqThrdLeHeatClimaLvlStrtReq : Outport  Port=28  OutDataTypeStr=Enum: TelmSeatClimaLvl  PortDimensions=1
  TelmBookSeatHeatClimalReqThrdRiHeatClimaLvlStrtReq_TelmBookSeatHeatClimalReqThrdRiHeatClimaLvlStrtReq : Outport  Port=29  OutDataTypeStr=Enum: TelmSeatClimaLvl  PortDimensions=1
  TelmBookSeatVentnClimalReqDrvVentnClimaLvlStrtReq_TelmBookSeatVentnClimalReqDrvVentnClimaLvlStrtReq : Outport  Port=30  OutDataTypeStr=Enum: TelmSeatClimaLvl  PortDimensions=1
  TelmBookSeatVentnClimalReqPassVentnClimaLvlStrtReq_TelmBookSeatVentnClimalReqPassVentnClimaLvlStrtReq : Outport  Port=31  OutDataTypeStr=Enum: TelmSeatClimaLvl  PortDimensions=1
  TelmBookSeatVentnClimalReqSecLeVentnClimaLvlStrtReq_TelmBookSeatVentnClimalReqSecLeVentnClimaLvlStrtReq : Outport  Port=32  OutDataTypeStr=Enum: TelmSeatClimaLvl  PortDimensions=1
  TelmBookSeatVentnClimalReqSecRiVentnClimaLvlStrtReq_TelmBookSeatVentnClimalReqSecRiVentnClimaLvlStrtReq : Outport  Port=33  OutDataTypeStr=Enum: TelmSeatClimaLvl  PortDimensions=1
  TelmBookSeatVentnClimalReqThrdLeVentnClimaLvlStrtReq_TelmBookSeatVentnClimalReqThrdLeVentnClimaLvlStrtReq : Outport  Port=34  OutDataTypeStr=Enum: TelmSeatClimaLvl  PortDimensions=1
  TelmBookSeatVentnClimalReqThrdRiVentnClimaLvlStrtReq_TelmBookSeatVentnClimalReqThrdRiVentnClimaLvlStrtReq : Outport  Port=35  OutDataTypeStr=Enum: TelmSeatClimaLvl  PortDimensions=1
  TelmBookSteerWhlHeatClimalReqStrtReq_TelmBookSteerWhlHeatClimalReqStrtReq : Outport  Port=36  OutDataTypeStr=Enum: OnOff1  PortDimensions=1
  TelmSeatVentnThrdReq_TelmSeatVentnThrdReq : Outport  Port=37  OutDataTypeStr=UInt32  PortDimensions=1
  UnLckClimaActvReqSt_UnLckClimaActvReqSt : Outport  Port=38  OutDataTypeStr=Boolean  PortDimensions=1
  UnLckHeatActvReqSt_UnLckHeatActvReqSt : Outport  Port=39  OutDataTypeStr=Boolean  PortDimensions=1
  ActvRqrdForPostPrkgClima_ActvRqrdForPostPrkgClima : Outport  Port=40  OutDataTypeStr=Boolean  PortDimensions=1
  ClimaOvrHeatProWarnIni_ClimaOvrHeatProWarnIni : Outport  Port=41  OutDataTypeStr=Enum: ClimaOvrheatProWarnSts  PortDimensions=1
  ClimaOvrHeatStrtReq_ClimaOvrHeatStrtReq : Outport  Port=42  OutDataTypeStr=Boolean  PortDimensions=1
  ClimaStrtTi_ClimaStrtTi : Outport  Port=43  OutDataTypeStr=CarTiGlb  PortDimensions=1
  CSDUsgChgeOffReq_CSDUsgChgeOffReq : Outport  Port=44  OutDataTypeStr=Boolean  PortDimensions=1
  CSDUsgChgeOnReq_CSDUsgChgeOnReq : Outport  Port=45  OutDataTypeStr=Boolean  PortDimensions=1
  EcuDogKeepActv_EcuDogKeepActv : Outport  Port=46  OutDataTypeStr=Boolean  PortDimensions=1
  EcuDogSlpReq_EcuDogSlpReq : Outport  Port=47  OutDataTypeStr=Boolean  PortDimensions=1
  EcuDogSlpSetTi_EcuDogSlpSetTi : Outport  Port=48  OutDataTypeStr=Ti33  PortDimensions=1
  ErsCmdByKeyStrtReq_ErsCmdByKeyStrtReq : Outport  Port=49  OutDataTypeStr=Enum: OnOff1  PortDimensions=1
  ErsRunTimelStrtReq_ErsRunTimelStrtReq : Outport  Port=50  OutDataTypeStr=Ti39  PortDimensions=1
  HeatrTypForPrkgClima_HeatrTypForPrkgClima : Outport  Port=51  OutDataTypeStr=Enum: HeatrTyp  PortDimensions=1
  HMIExtVentFanVolReqStrtReq_HMIExtVentFanVolReqStrtReq : Outport  Port=52  OutDataTypeStr=Enum: FanVolReq  PortDimensions=1
  HMIExtVentonoffReqStrtReq_HMIExtVentonoffReqStrtReq : Outport  Port=53  OutDataTypeStr=Enum: OnOffNoReq  PortDimensions=1
  HMIExtVentTSpReqStrtReq_HMIExtVentTSpReqStrtReq : Outport  Port=54  OutDataTypeStr=Enum: TSpReq  PortDimensions=1
  HMIExtVentTStsReqStrtReq_HMIExtVentTStsReqStrtReq : Outport  Port=55  OutMin=-256  OutMax=255.9  OutDataTypeStr=T2  PortDimensions=1
  HvBattForPrkgClima_HvBattForPrkgClima : Outport  Port=56  OutDataTypeStr=Boolean  PortDimensions=1
  IntCo2MontrStrtReq_IntCo2MontrStrtReq : Outport  Port=57  OutDataTypeStr=Boolean  PortDimensions=1
  NetReqFromPrkgClimaEveMgr_NetReqFromPrkgClimaEveMgr : Outport  Port=58  OutDataTypeStr=Boolean  PortDimensions=1
  ObsvrActvnReqFromPrkgClima_ObsvrActvnReqFromPrkgClima : Outport  Port=59  OutDataTypeStr=Boolean  PortDimensions=1
  OffRqrdForPrkgClima_OffRqrdForPrkgClima : Outport  Port=60  OutDataTypeStr=Boolean  PortDimensions=1
  OffRqrdForRemClima_OffRqrdForRemClima : Outport  Port=61  OutDataTypeStr=Boolean  PortDimensions=1
  PostDrvgClimaAvl_Ngcc_PostDrvgClimaAvl : Outport  Port=62  OutDataTypeStr=Enum: OnOff1  PortDimensions=1
  PrePrkgClimaNotAvlForHvSysWoU_PrePrkgClimaNotAvlForHvSysWoU : Outport  Port=63  OutDataTypeStr=Boolean  PortDimensions=1
  PrkgCDSAvl_PrkgCDSAvl : Outport  Port=64  OutDataTypeStr=Boolean  PortDimensions=1
  PrkgClimaAllwdForBattULo_PrkgClimaAllwdForBattULo : Outport  Port=65  OutDataTypeStr=Boolean  PortDimensions=1
  PrkgClimaAllwdForSwtHeatrTyp_PrkgClimaAllwdForSwtHeatrTyp : Outport  Port=66  OutDataTypeStr=Boolean  PortDimensions=1
  RemClimaallExsrcReq_RemClimaallExsrcReq : Outport  Port=67  OutDataTypeStr=UInt32  PortDimensions=1
  RemClimaEgyExsrcReq_RemClimaEgyExsrcReq : Outport  Port=68  OutDataTypeStr=UInt32  PortDimensions=1
  RemClimaTmrStrtReq_RemClimaTmrStrtReq : Outport  Port=69  OutDataTypeStr=Ti33  PortDimensions=1
  RemStrtExtnTiStrtReq_RemStrtExtnTiStrtReq : Outport  Port=70  OutDataTypeStr=Ti39  PortDimensions=1
  RemStrtHvCtrlStrtReq_RemStrtHvCtrlStrtReq : Outport  Port=71  OutDataTypeStr=Enum: ErsCmd  PortDimensions=1
  RemVentReqStrtReq_RemVentReqStrtReq : Outport  Port=72  OutDataTypeStr=Boolean  PortDimensions=1
  ReqForSwtHeatrTypFromEveMgr_ReqForSwtHeatrTypFromEveMgr : Outport  Port=73  OutDataTypeStr=Boolean  PortDimensions=1
  RtBasSdlForTmrPrePrkgClima_RtBasSdlForTmrPrePrkgClima : Outport  Port=74  OutDataTypeStr=Ti33  PortDimensions=1
  RtCmftForImdtPrePrkgClima_RtCmftForImdtPrePrkgClima : Outport  Port=75  OutDataTypeStr=Ti33  PortDimensions=1
  RtCmftForPostPrkgClima_RtCmftForPostPrkgClima : Outport  Port=76  OutDataTypeStr=Ti33  PortDimensions=1
  RtCmftForPreClng_RtCmftForPreClng : Outport  Port=77  OutDataTypeStr=Ti33  PortDimensions=1
  RtCmftForTmrPrePrkgClima_RtCmftForTmrPrePrkgClima : Outport  Port=78  OutDataTypeStr=Ti33  PortDimensions=1
  RtMaxForPrePrkgClima_RtMaxForPrePrkgClima : Outport  Port=79  OutDataTypeStr=Ti33  PortDimensions=1
  SeatHeatForPrkgClimaEnad_SeatHeatForPrkgClimaEnad : Outport  Port=80  OutDataTypeStr=Enum: SeatHeatDurgClimaEnad  PortDimensions=1
  SteerWhlHeatgForPrkgClimaEnad_SteerWhlHeatgForPrkgClimaEnad : Outport  Port=81  OutDataTypeStr=Boolean  PortDimensions=1
  StrtRqrdForDirForPrePrkgClima_StrtRqrdForDirForPrePrkgClima : Outport  Port=82  OutDataTypeStr=Boolean  PortDimensions=1
  StrtRqrdForPreClng_StrtRqrdForPreClng : Outport  Port=83  OutDataTypeStr=Boolean  PortDimensions=1
  StrtRqrdForTmrForPrePrkgClima_StrtRqrdForTmrForPrePrkgClima : Outport  Port=84  OutDataTypeStr=Boolean  PortDimensions=1
  SuspStrtAllwdForPrkgClima_SuspStrtAllwdForPrkgClima : Outport  Port=85  OutDataTypeStr=Boolean  PortDimensions=1
  TelmClimaExtVentnLvlStrtReq_TelmClimaExtVentnLvlStrtReq : Outport  Port=86  OutDataTypeStr=Enum: HmiHvacFanLvl  PortDimensions=1
  TelmClimaExtVentnReqStrtReq_TelmClimaExtVentnReqStrtReq : Outport  Port=87  OutDataTypeStr=Enum: OnOffNoReq  PortDimensions=1
  TelmClimaExtVentnTempRangeStrtReq_TelmClimaExtVentnTempRangeStrtReq : Outport  Port=88  OutMin=-256  OutMax=255.9  OutDataTypeStr=T2  PortDimensions=1
  TelmClimaExtVentnTSpSpclStrtReq_TelmClimaExtVentnTSpSpclStrtReq : Outport  Port=89  OutDataTypeStr=Enum: HmiCmptmtTSpSpcl  PortDimensions=1
  UpdRtTotForTmrForPrePrkgClima_UpdRtTotForTmrForPrePrkgClima : Outport  Port=90  OutDataTypeStr=Boolean  PortDimensions=1
  WarnForHybWoU_WarnForHybWoU : Outport  Port=91  OutDataTypeStr=Boolean  PortDimensions=1
  WarnForHybWoUForTmr_WarnForHybWoUForTmr : Outport  Port=92  OutDataTypeStr=Boolean  PortDimensions=1
  WarnForPreCdngFctNotAvl_WarnForPreCdngFctNotAvl : Outport  Port=93  OutDataTypeStr=Boolean  PortDimensions=1
  WarnForTmrPreCdngFctNotAvl_WarnForTmrPreCdngFctNotAvl : Outport  Port=94  OutDataTypeStr=Boolean  PortDimensions=1
  IntCo2SetStartTime_IntCo2SetStartTime : Outport  Port=95  OutMin=0  OutMax=59  OutDataTypeStr=Mins1  PortDimensions=1
  IntCo2SetStopTime_IntCo2SetStopTime : Outport  Port=96  OutMin=0  OutMax=59  OutDataTypeStr=Mins1  PortDimensions=1
  RemClimaTempStrtReq_RemClimaTempStrtReq : Outport  Port=97  OutMin=-256  OutMax=255.9  OutDataTypeStr=T2  PortDimensions=1
  wires:
  AmbTFildForClimaNgcc_AmbTFildForClima/1 -> PrkgClimaEveMgr_Runnable_sys/1(AmbTFildForClimaNgcc_AmbTFildForClima_read)
  BookTrvlClimaActvReqfromExsrc_BookTrvlClimaActvReqfromExsrc/1 -> PrkgClimaEveMgr_Runnable_sys/2(BookTrvlClimaActvReqfromExsrc_BookTrvlClimaActvReqfromExsrc_read)
  CarTiIntForClima_CarTiIntForClima/1 -> PrkgClimaEveMgr_Runnable_sys/3(CarTiIntForClima_CarTiIntForClima_read)
  ClimaCfgForCds1_ClimaCfgForCds1/1 -> PrkgClimaEveMgr_Runnable_sys/4(ClimaCfgForCds1_ClimaCfgForCds1_read)
  ClimaCfgForClimaCtrlMan1_ClimaCfgForClimaCtrlMan1/1 -> PrkgClimaEveMgr_Runnable_sys/5(ClimaCfgForClimaCtrlMan1_ClimaCfgForClimaCtrlMan1_read)
  ClimaCfgForCoolgPreCdn1_ClimaCfgForCoolgPreCdn1/1 -> PrkgClimaEveMgr_Runnable_sys/6(ClimaCfgForCoolgPreCdn1_ClimaCfgForCoolgPreCdn1_read)
  ClimaCfgForCooltCircForClima1_ClimaCfgForCooltCircForClima1/1 -> PrkgClimaEveMgr_Runnable_sys/7(ClimaCfgForCooltCircForClima1_ClimaCfgForCooltCircForClima1_read)
  ClimaCfgForEcuCooltVlvOutp1_ClimaCfgForEcuCooltVlvOutp1/1 -> PrkgClimaEveMgr_Runnable_sys/8(ClimaCfgForEcuCooltVlvOutp1_ClimaCfgForEcuCooltVlvOutp1_read)
  ClimaCfgForExtVentnClimaCtrl_ClimaCfgForExtVentnClimaCtrl/1 -> PrkgClimaEveMgr_Runnable_sys/9(ClimaCfgForExtVentnClimaCtrl_ClimaCfgForExtVentnClimaCtrl_read)
  ClimaCfgForFoldCSD_ClimaCfgForFoldCSD/1 -> PrkgClimaEveMgr_Runnable_sys/10(ClimaCfgForFoldCSD_ClimaCfgForFoldCSD_read)
  ClimaCfgForForRemEngStrtPreCdn_ClimaCfgForForRemEngStrtPreCdn/1 -> PrkgClimaEveMgr_Runnable_sys/11(ClimaCfgForForRemEngStrtPreCdn_ClimaCfgForForRemEngStrtPreCdn_read)
  ClimaCfgForHeatgPreCdnAvl1_ClimaCfgForHeatgPreCdnAvl1/1 -> PrkgClimaEveMgr_Runnable_sys/12(ClimaCfgForHeatgPreCdnAvl1_ClimaCfgForHeatgPreCdnAvl1_read)
  ClimaCfgForHeatrTyp1_ClimaCfgForHeatrTyp1/1 -> PrkgClimaEveMgr_Runnable_sys/13(ClimaCfgForHeatrTyp1_ClimaCfgForHeatrTyp1_read)
  ClimaCfgForHvacHeatrElecFrntPrsnt1_ClimaCfgForHvacHeatrElecFrntPrsnt1/1 -> PrkgClimaEveMgr_Runnable_sys/14(ClimaCfgForHvacHeatrElecFrntPrsnt1_ClimaCfgForHvacHeatrElecFrntPrsnt1_read)